simulink library "inp"  (Simulink 8.2)
settings: Solver=ode45  SolverMode=SingleTasking  StartTime=0.0  StopTime=10.0  RelTol=1e-3
undefined symbols (not defined in the file or in its folder): C_TICK_TIME_S, ENUM_FAULTSTATE_FAIL, ENUM_FAULTSTATE_INDETERMINATE, ENUM_FAULTSTATE_PASS, ENUM_KEYSWITCH_ON, FALSE, KINP_ABS1CANCommuOk_flg_ovrdflg, KINP_ABS1CANCommuOk_flg_ovrdval, KINP_ABS1CANPeriodTime_s, KINP_ABS1CANRolCntOk_flg_ovrdflg, KINP_ABS1CANRolCntOk_flg_ovrdval, KINP_ABS2CANChkSumOk_flg_ovrdflg, KINP_ABS2CANChkSumOk_flg_ovrdval, KINP_ABS2CANCommuOk_flg_ovrdflg, KINP_ABS2CANCommuOk_flg_ovrdval, KINP_ABS2CANPeriodTime_s, KINP_ABS2CANRolCntOk_flg_ovrdflg, KINP_ABS2CANRolCntOk_flg_ovrdval, KINP_ABS3CANCommuOk_flg_ovrdflg, KINP_ABS3CANCommuOk_flg_ovrdval, KINP_ABS4CANCommuOk_flg_ovrdflg, KINP_ABS4CANCommuOk_flg_ovrdval, KINP_ABSActive_flg_defval, KINP_ABSActive_flg_invalid, KINP_ABSActive_flg_ovrdflg, KINP_ABSActive_flg_ovrdval, KINP_ABSActive_flg_setvalid, KINP_ABSActive_flg_srvcflg, KINP_ABSActive_flg_srvcval, KINP_ABSCANAlive_flg_ovrdflg, KINP_ABSCANAlive_flg_ovrdval, KINP_ABSCANCommuOk_flg_ovrdflg, KINP_ABSCANCommuOk_flg_ovrdval, KINP_ABSCANValidOk_flg_ovrdflg, KINP_ABSCANValidOk_flg_ovrdval, KINP_ABSFault_flg_defval, KINP_ABSFault_flg_invalid, KINP_ABSFault_flg_ovrdflg, KINP_ABSFault_flg_ovrdval, KINP_ABSFault_flg_setvalid, KINP_ABSFault_flg_srvcflg, KINP_ABSFault_flg_srvcval, KINP_AC1CANCommuOk_flg_ovrdflg, KINP_AC1CANCommuOk_flg_ovrdval, KINP_ACCANAlive_flg_ovrdflg, KINP_ACCANAlive_flg_ovrdval, KINP_ACCANCommuOk_flg_ovrdflg, KINP_ACCANCommuOk_flg_ovrdval, KINP_ACCANValidOk_flg_ovrdflg, KINP_ACCANValidOk_flg_ovrdval, KINP_ACCCancelH_flg_defval, KINP_ACCCancelH_flg_invalid, KINP_ACCCancelH_flg_ovrdflg, KINP_ACCCancelH_flg_ovrdval, KINP_ACCCancelH_flg_setvalid, KINP_ACCCancelH_flg_srvcflg, KINP_ACCCancelH_flg_srvcval, KINP_AccelPedalPosn_pct_defval, KINP_AccelPedalPosn_pct_gain, KINP_AccelPedalPosn_pct_invalid ... (+1866)
libraries: inp x192, hcu_lib x34, simulink x33
(part 21 of 21)

== system inp/INP/InputSignals/SignalProcess/Proc_HMIButtons ==
  VINP_LFCPilotOnH_flg_InHAL : Inport
  VINP_LFCPilotCancelH_flg_InHAL : Inport  Port=2
  VINP_ACCOnH_flg_InHAL : Inport  Port=3
  VINP_ACCCancelH_flg_InHAL : Inport  Port=4
  VINP_ACCResSpdIncH_flg_InHAL : Inport  Port=5
  VINP_ACCSetSpdDecH_flg_InHAL : Inport  Port=6
  VINP_ACCSpdIncH_flg_InHAL : Inport  Port=7
  VINP_ACCSpdDecH_flg_InHAL : Inport  Port=8
  VINP_ACCTimeGapIncH_flg_InHAL : Inport  Port=9
  VINP_ACCTimeGapDecH_flg_InHAL : Inport  Port=10
  VINP_IGSAOnH_flg_InHAL : Inport  Port=11
  VINP_IGSAResSpdIncH_flg_InHAL : Inport  Port=12
  VINP_IGSASetSpdDecH_flg_InHAL : Inport  Port=13
  VINP_IGSASwitchModeH_flg_InHAL : Inport  Port=14
  Detect Change{,1..13} (14 alike) : simulink/Logic and Bit Operations/Detect Change  vinit=0  InputProcessing=Inherited  OutDataTypeStr=uint8
  VINP_LFCPilotOnH_flg_InProc : Outport
  VINP_LFCPilotCancelH_flg_InProc : Outport  Port=2
  VINP_ACCOnH_flg_InProc : Outport  Port=3
  VINP_ACCCancelH_flg_InProc : Outport  Port=4
  VINP_ACCResSpdIncH_flg_InProc : Outport  Port=5
  VINP_ACCSetSpdDecH_flg_InProc : Outport  Port=6
  VINP_ACCSpdIncH_flg_InProc : Outport  Port=7
  VINP_ACCSpdDecH_flg_InProc : Outport  Port=8
  VINP_ACCTimeGapIncH_flg_InProc : Outport  Port=9
  VINP_ACCTimeGapDecH_flg_InProc : Outport  Port=10
  VINP_IGSAOnH_flg_InProc : Outport  Port=11
  VINP_IGSAResSpdIncH_flg_InProc : Outport  Port=12
  VINP_IGSASetSpdDecH_flg_InProc : Outport  Port=13
  VINP_IGSASwitchModeH_flg_InProc : Outport  Port=14
  wires:
  VINP_LFCPilotOnH_flg_InHAL/1 -> Detect Change/1
  Detect Change/1 -> VINP_LFCPilotOnH_flg_InProc/1  "VINP_LFCPilotOnH_flg_InProc"
  VINP_LFCPilotCancelH_flg_InHAL/1 -> Detect Change1/1
  Detect Change1/1 -> VINP_LFCPilotCancelH_flg_InProc/1  "VINP_LFCPilotCancelH_flg_InProc"
  VINP_ACCOnH_flg_InHAL/1 -> Detect Change2/1
  Detect Change2/1 -> VINP_ACCOnH_flg_InProc/1  "VINP_ACCOnH_flg_InProc"
  VINP_ACCCancelH_flg_InHAL/1 -> Detect Change3/1
  Detect Change3/1 -> VINP_ACCCancelH_flg_InProc/1  "VINP_ACCCancelH_flg_InProc"
  VINP_ACCResSpdIncH_flg_InHAL/1 -> Detect Change4/1
  Detect Change4/1 -> VINP_ACCResSpdIncH_flg_InProc/1  "VINP_ACCResSpdIncH_flg_InProc"
  VINP_ACCSetSpdDecH_flg_InHAL/1 -> Detect Change5/1
  Detect Change5/1 -> VINP_ACCSetSpdDecH_flg_InProc/1  "VINP_ACCSetSpdDecH_flg_InProc"
  VINP_ACCSpdIncH_flg_InHAL/1 -> Detect Change6/1
  Detect Change6/1 -> VINP_ACCSpdIncH_flg_InProc/1  "VINP_ACCSpdIncH_flg_InProc"
  VINP_ACCSpdDecH_flg_InHAL/1 -> Detect Change7/1
  Detect Change7/1 -> VINP_ACCSpdDecH_flg_InProc/1  "VINP_ACCSpdDecH_flg_InProc"
  VINP_ACCTimeGapIncH_flg_InHAL/1 -> Detect Change8/1
  Detect Change8/1 -> VINP_ACCTimeGapIncH_flg_InProc/1  "VINP_ACCTimeGapIncH_flg_InProc"
  VINP_ACCTimeGapDecH_flg_InHAL/1 -> Detect Change9/1
  Detect Change9/1 -> VINP_ACCTimeGapDecH_flg_InProc/1  "VINP_ACCTimeGapDecH_flg_InProc"
  VINP_IGSAOnH_flg_InHAL/1 -> Detect Change10/1
  Detect Change10/1 -> VINP_IGSAOnH_flg_InProc/1  "VINP_IGSAOnH_flg_InProc"
  VINP_IGSAResSpdIncH_flg_InHAL/1 -> Detect Change11/1
  Detect Change11/1 -> VINP_IGSAResSpdIncH_flg_InProc/1  "VINP_IGSAResSpdIncH_flg_InProc"
  VINP_IGSASetSpdDecH_flg_InHAL/1 -> Detect Change12/1
  Detect Change12/1 -> VINP_IGSASetSpdDecH_flg_InProc/1  "VINP_IGSASetSpdDecH_flg_InProc"
  VINP_IGSASwitchModeH_flg_InHAL/1 -> Detect Change13/1
  Detect Change13/1 -> VINP_IGSASwitchModeH_flg_InProc/1  "VINP_IGSASwitchModeH_flg_InProc"

== system inp/INP/InputSignals/SignalProcess/Proc_IgnitionActive ==
  VINP_IgnitionVolt_V_InHAL : Inport
  VINP_KeySwitchStatus_enum_InHAL : Inport  Port=2
  Constant : Constant  Value=ENUM_KEYSWITCH_ON
  Constant1 : Constant  Value=KINP_IgnitionActiveVolt_V
  Logical Operator : Logic  Operator=OR  AllPortsSameDT=off  OutDataTypeStr=boolean
  Relational Operator : RelationalOperator  Operator===  OutDataTypeStr=boolean
  Relational Operator1 : RelationalOperator  Operator=>  OutDataTypeStr=boolean
  VINP_IgnitionActive_flg_InProc : Outport
  wires:
  Constant1/1 -> Relational Operator1/2
  Relational Operator1/1 -> Logical Operator/1
  VINP_IgnitionVolt_V_InHAL/1 -> Relational Operator1/1
  Constant/1 -> Relational Operator/2
  Relational Operator/1 -> Logical Operator/2
  VINP_KeySwitchStatus_enum_InHAL/1 -> Relational Operator/1
  Logical Operator/1 -> VINP_IgnitionActive_flg_InProc/1  "VINP_IgnitionActive_flg_InProc"

== system inp/INP/InputSignals/SignalProcess/Proc_LaneEstimator ==
  VINP_AppTime_ms_InProc : Inport
  Task_Time_s : Inport  Port=2
  VINP_YawRate_radps_InHAL : Inport  Port=3
  VINP_VehSpd_mps_InHAL : Inport  Port=4
  VINP_LaneC0L_m_InHAL : Inport  Port=5
  VINP_LaneC1L_rad_InHAL : Inport  Port=6
  VINP_LaneC2L_rat_InHAL : Inport  Port=7
  VINP_LaneC3L_rat_InHAL : Inport  Port=8
  VINP_LaneC0R_m_InHAL : Inport  Port=9
  VINP_LaneC1R_rad_InHAL : Inport  Port=10
  VINP_LaneC2R_rat_InHAL : Inport  Port=11
  VINP_LaneC3R_rat_InHAL : Inport  Port=12
  VINP_LaneQualityL_enum_InHAL : Inport  Port=13
  VINP_LaneQualityR_enum_InHAL : Inport  Port=14
  Ground{,1..7} (8 alike) : Ground
  Terminator{,1..13} (14 alike) : Terminator
  VINP_LaneC0LKalF_m_InProc : Outport
  VINP_LaneC1LKalF_rad_InProc : Outport  Port=2
  VINP_LaneC2LKalF_rat_InProc : Outport  Port=3
  VINP_LaneC3LKalF_rat_InProc : Outport  Port=4
  VINP_LaneC0RKalF_m_InProc : Outport  Port=5
  VINP_LaneC1RKalF_rad_InProc : Outport  Port=6
  VINP_LaneC2RKalF_rat_InProc : Outport  Port=7
  VINP_LaneC3RKalF_rat_InProc : Outport  Port=8
  wires:
  Ground6/1 -> VINP_LaneC3LKalF_rat_InProc/1  "VINP_LaneC3LKalF_rat_InProc"
  Ground7/1 -> VINP_LaneC3RKalF_rat_InProc/1  "VINP_LaneC3RKalF_rat_InProc"
  Ground/1 -> VINP_LaneC0LKalF_m_InProc/1  "VINP_LaneC0LKalF_m_InProc"
  Ground1/1 -> VINP_LaneC1LKalF_rad_InProc/1  "VINP_LaneC1LKalF_rad_InProc"
  Ground2/1 -> VINP_LaneC2LKalF_rat_InProc/1  "VINP_LaneC2LKalF_rat_InProc"
  Ground3/1 -> VINP_LaneC0RKalF_m_InProc/1  "VINP_LaneC0RKalF_m_InProc"
  Ground4/1 -> VINP_LaneC1RKalF_rad_InProc/1  "VINP_LaneC1RKalF_rad_InProc"
  Ground5/1 -> VINP_LaneC2RKalF_rat_InProc/1  "VINP_LaneC2RKalF_rat_InProc"
  VINP_AppTime_ms_InProc/1 -> Terminator/1
  Task_Time_s/1 -> Terminator1/1
  VINP_YawRate_radps_InHAL/1 -> Terminator2/1
  VINP_VehSpd_mps_InHAL/1 -> Terminator3/1
  VINP_LaneQualityL_enum_InHAL/1 -> Terminator4/1
  VINP_LaneQualityR_enum_InHAL/1 -> Terminator5/1
  VINP_LaneC0L_m_InHAL/1 -> Terminator6/1
  VINP_LaneC1L_rad_InHAL/1 -> Terminator7/1
  VINP_LaneC2L_rat_InHAL/1 -> Terminator8/1
  VINP_LaneC3L_rat_InHAL/1 -> Terminator9/1
  VINP_LaneC0R_m_InHAL/1 -> Terminator10/1
  VINP_LaneC1R_rad_InHAL/1 -> Terminator11/1
  VINP_LaneC2R_rat_InHAL/1 -> Terminator12/1
  VINP_LaneC3R_rat_InHAL/1 -> Terminator13/1

== system inp/INP/InputSignals/SignalProcess/Proc_OtherHMISwitches ==
  VINP_RearFogSwOn_flg_InHAL : Inport
  VINP_RearFogSwOnValid_flg_InHAL : Inport  Port=2
  Data Type Conversion : DataTypeConversion  OutDataTypeStr=boolean
  RearFogOnSwitchLatch : Stateflow chart  TreatAsAtomicUnit=on
  VINP_RearFogSwOn_flg_InProc : Outport
  VINP_RearFogSwOnValid_flg_InProc : Outport  Port=2
  wires:
  VINP_RearFogSwOn_flg_InHAL/1 -> RearFogOnSwitchLatch/1(VINP_RearFogSwOn_flg_InHAL)
  RearFogOnSwitchLatch/1(VINP_RearFogSwOn_flg_InProc) -> VINP_RearFogSwOn_flg_InProc/1
  Data Type Conversion/1 -> VINP_RearFogSwOnValid_flg_InProc/1  "VINP_RearFogSwOnValid_flg_InProc"
  VINP_RearFogSwOnValid_flg_InHAL/1 -> Data Type Conversion/1

== system inp/INP/InputSignals/SignalProcess/Proc_SteerAngleUnit ==
  VINP_SteerAngle_deg_InHAL : Inport
  deg2rad : hcu_lib/deg2rad
  VINP_SteerAngle_rad_InProc : Outport
  wires:
  VINP_SteerAngle_deg_InHAL/1 -> deg2rad/1
  deg2rad/1 -> VINP_SteerAngle_rad_InProc/1  "VINP_SteerAngle_rad_InProc"

== system inp/INP/InputSignals/SignalProcess/Proc_SteerSpeedCal ==
  VINP_SteerAngle_deg_InHAL : Inport
  VINP_SteerAngleValid_flg_InHAL : Inport  Port=2
  CAN_Update_flag : Stateflow chart  TreatAsAtomicUnit=on
  Data Type Conversion : DataTypeConversion
  Detect Change : simulink/Logic and Bit Operations/Detect Change  vinit=0  InputProcessing=Inherited  OutDataTypeStr=boolean
  SteerSpeedCalculation : Subsystem
  SteerSpeedCalculation1 : Subsystem
  StrRate_SW : Constant  Value=0  OutDataTypeStr=boolean
  StrRate_calculation : Stateflow chart  TreatAsAtomicUnit=on
  Switch : Switch
  Trigger signal generator : Subsystem  TreatAsAtomicUnit=on
  Unit Delay1 : UnitDelay  SampleTime=-1
  VINP_SteerSpeed_degps_InProc : Outport
  VINP_SteerSpeedValid_flg_InProc : Outport  Port=2
  wires:
  Trigger signal generator/1(Trigger_signal) -> SteerSpeedCalculation1/Trigger, SteerSpeedCalculation/Trigger
  Data Type Conversion/1 -> VINP_SteerSpeedValid_flg_InProc/1  "VINP_SteerSpeedValid_flg_InProc"
  VINP_SteerAngle_deg_InHAL/1 -> SteerSpeedCalculation1/1(VSTR_StrAngReq_deg), SteerSpeedCalculation/1(In1)
  VINP_SteerAngleValid_flg_InHAL/1 -> Data Type Conversion/1
  StrRate_calculation/1(StrRate) -> Switch/3, Unit Delay1/1
  CAN_Update_flag/1(CAN_flag) -> StrRate_calculation/1(CAN_flag)  "CAN_flag"
  Detect Change/1 -> CAN_Update_flag/1(AngleChg)
  SteerSpeedCalculation/1(StrAng) -> Detect Change/1, StrRate_calculation/2(StrAng)  "StrAng"
  SteerSpeedCalculation/2(StrAng1) -> StrRate_calculation/3(StrAng1)  "StrAng1"
  SteerSpeedCalculation/3(StrAng2) -> StrRate_calculation/4(StrAng2)  "StrAng2"
  SteerSpeedCalculation/4(StrAng3) -> StrRate_calculation/5(StrAng3)  "StrAng3"
  SteerSpeedCalculation/5(StrAng4) -> StrRate_calculation/6(StrAng4)  "StrAng4"
  Unit Delay1/1 -> StrRate_calculation/7(StrRateLast)  "StrRateLast"
  Switch/1 -> VINP_SteerSpeed_degps_InProc/1  "VINP_SteerSpeed_degps_InProc"
  SteerSpeedCalculation1/1(VSTR_StrRateReq_degps) -> Switch/1
  StrRate_SW/1 -> Switch/2

== system inp/INP/InputSignals/SignalProcess/Proc_SteeringWheelButtons ==
  VINP_ACCOnVCU_flg_InHAL : Inport
  VINP_ACCOnVCUValid_flg_InHAL : Inport  Port=2
  VINP_ACCTimeGapDecVCU_flg_InHAL : Inport  Port=3
  VINP_ACCTimeGapDecVCUValid_flg_InHAL : Inport  Port=4
  VINP_LFCOnVCU_flg_InHAL : Inport  Port=5
  VINP_LFCOnVCUValid_flg_InHAL : Inport  Port=6
  Data Type Conversion{,1..3} (4 alike) : DataTypeConversion  OutDataTypeStr=boolean
  Detect Increase : simulink/Logic and Bit Operations/Detect Increase  vinit=0.0  InputProcessing=Inherited  OutDataTypeStr=boolean
  Detect Increase1 : simulink/Logic and Bit Operations/Detect Increase  vinit=0.0  InputProcessing=Inherited  OutDataTypeStr=boolean
  VINP_ACCOnVCU_flg_InProc : Outport
  VINP_ACCOnVCUValid_flg_InProc : Outport  Port=2
  VINP_ACCDistVCU_flg_InProc : Outport  Port=3
  VINP_ACCDistVCUValid_flg_InProc : Outport  Port=4
  VINP_LFCOnVCU_flg_InProc : Outport  Port=5
  VINP_LFCOnVCUValid_flg_InProc : Outport  Port=6
  wires:
  Data Type Conversion/1 -> VINP_ACCOnVCUValid_flg_InProc/1  "VINP_ACCOnVCUValid_flg_InProc"
  VINP_ACCOnVCUValid_flg_InHAL/1 -> Data Type Conversion/1
  Detect Increase1/1 -> VINP_ACCOnVCU_flg_InProc/1  "VINP_ACCOnVCU_flg_InProc"
  VINP_ACCOnVCU_flg_InHAL/1 -> Detect Increase1/1
  VINP_ACCTimeGapDecVCU_flg_InHAL/1 -> Data Type Conversion1/1
  VINP_ACCTimeGapDecVCUValid_flg_InHAL/1 -> Data Type Conversion2/1
  Data Type Conversion1/1 -> VINP_ACCDistVCU_flg_InProc/1  "VINP_ACCDistVCU_flg_InProc"
  Data Type Conversion2/1 -> VINP_ACCDistVCUValid_flg_InProc/1  "VINP_ACCDistVCUValid_flg_InProc"
  Data Type Conversion3/1 -> VINP_LFCOnVCUValid_flg_InProc/1  "VINP_LFCOnVCUValid_flg_InProc"
  VINP_LFCOnVCUValid_flg_InHAL/1 -> Data Type Conversion3/1
  Detect Increase/1 -> VINP_LFCOnVCU_flg_InProc/1  "VINP_LFCOnVCU_flg_InProc"
  VINP_LFCOnVCU_flg_InHAL/1 -> Detect Increase/1

== system inp/INP/InputSignals/SignalProcess/Proc_VehicleSpeedUnit ==
  VINP_VehSpd_kph_InHAL : Inport
  kph2mps : hcu_lib/kph2mps
  VINP_VehSpd_mps_InProc : Outport
  wires:
  kph2mps/1 -> VINP_VehSpd_mps_InProc/1  "VINP_VehSpd_mps_InProc"
  VINP_VehSpd_kph_InHAL/1 -> kph2mps/1

== system inp/INP/InputSignals/SignalProcess/Proc_YawRateUnit ==
  VINP_YawRate_degps_InHAL : Inport
  deg2rad : hcu_lib/deg2rad
  VINP_YawRate_radps_InProc : Outport
  wires:
  deg2rad/1 -> VINP_YawRate_radps_InProc/1  "VINP_YawRate_radps_InProc"
  VINP_YawRate_degps_InHAL/1 -> deg2rad/1

== system inp/INP/METER_signals/MeterVehSpd/conv_gain_offset ==
  in : Inport
  Product : Product  RndMeth=Zero
  Sum : Sum
  gain : Constant  Value=KINP_MeterVehSpd_kph_gain
  offset : Constant  Value=KINP_MeterVehSpd_kph_offset
  out : Outport
  wires:
  offset/1 -> Sum/2
  Product/1 -> Sum/1
  gain/1 -> Product/2
  in/1 -> Product/1
  Sum/1 -> out/1

== system inp/INP/METER_signals/MeterVehSpd/min_max_limit ==
  in : Inport
  MinMax10 : MinMax  Function=max  Inputs=2
  MinMax5 : MinMax  Inputs=2
  maxval : Constant  Value=KINP_MeterVehSpd_kph_maxval
  minval : Constant  Value=KINP_MeterVehSpd_kph_minval
  out : Outport
  wires:
  minval/1 -> MinMax10/2
  maxval/1 -> MinMax5/2
  MinMax10/1 -> out/1
  in/1 -> MinMax5/1
  MinMax5/1 -> MinMax10/1

== system inp/INP/METER_signals/OdoMeter/conv_gain_offset ==
  in : Inport
  Product : Product  RndMeth=Zero
  Sum : Sum
  gain : Constant  Value=KINP_OdoMeter_km_gain
  offset : Constant  Value=KINP_OdoMeter_km_offset
  out : Outport
  wires:
  Sum/1 -> out/1
  in/1 -> Product/1
  gain/1 -> Product/2
  Product/1 -> Sum/1
  offset/1 -> Sum/2

== system inp/INP/METER_signals/OdoMeter/min_max_limit ==
  in : Inport
  MinMax10 : MinMax  Function=max  Inputs=2
  MinMax5 : MinMax  Inputs=2
  maxval : Constant  Value=KINP_OdoMeter_km_maxval
  minval : Constant  Value=KINP_OdoMeter_km_minval
  out : Outport
  wires:
  MinMax5/1 -> MinMax10/1
  in/1 -> MinMax5/1
  MinMax10/1 -> out/1
  maxval/1 -> MinMax5/2
  minval/1 -> MinMax10/2

== system inp/INP/TCM_signals/TMTurbineSpd/conv_gain_offset ==
  in : Inport
  Product : Product  RndMeth=Zero
  Sum : Sum
  gain : Constant  Value=KINP_TMTurbineSpd_rpm_gain
  offset : Constant  Value=KINP_TMTurbineSpd_rpm_offset
  out : Outport
  wires:
  Sum/1 -> out/1
  in/1 -> Product/1
  gain/1 -> Product/2
  Product/1 -> Sum/1
  offset/1 -> Sum/2

== system inp/INP/TCM_signals/TMTurbineSpd/min_max_limit ==
  in : Inport
  MinMax10 : MinMax  Function=max  Inputs=2
  MinMax5 : MinMax  Inputs=2
  maxval : Constant  Value=KINP_TMTurbineSpd_rpm_maxval
  minval : Constant  Value=KINP_TMTurbineSpd_rpm_minval
  out : Outport
  wires:
  MinMax5/1 -> MinMax10/1
  in/1 -> MinMax5/1
  MinMax10/1 -> out/1
  maxval/1 -> MinMax5/2
  minval/1 -> MinMax10/2

== system inp/INP/VCU_signals/AckCurBrakDecReq/conv_gain_offset ==
  in : Inport
  Product : Product  RndMeth=Zero
  Sum : Sum
  gain : Constant  Value=KINP_AckCurBrakDecReq_mps2_gain
  offset : Constant  Value=KINP_AckCurBrakDecReq_mps2_offset
  out : Outport
  wires:
  Sum/1 -> out/1
  in/1 -> Product/1
  gain/1 -> Product/2
  Product/1 -> Sum/1
  offset/1 -> Sum/2

== system inp/INP/VCU_signals/AckCurBrakDecReq/min_max_limit ==
  in : Inport
  MinMax10 : MinMax  Function=max  Inputs=2
  MinMax5 : MinMax  Inputs=2
  maxval : Constant  Value=KINP_AckCurBrakDecReq_mps2_maxval
  minval : Constant  Value=KINP_AckCurBrakDecReq_mps2_minval
  out : Outport
  wires:
  MinMax5/1 -> MinMax10/1
  in/1 -> MinMax5/1
  MinMax10/1 -> out/1
  maxval/1 -> MinMax5/2
  minval/1 -> MinMax10/2

== system inp/INP/VCU_signals/AckCurPTTqReq/conv_gain_offset ==
  in : Inport
  Product : Product  RndMeth=Zero
  Sum : Sum
  gain : Constant  Value=KINP_AckCurPTTqReq_Nm_gain
  offset : Constant  Value=KINP_AckCurPTTqReq_Nm_offset
  out : Outport
  wires:
  offset/1 -> Sum/2
  Product/1 -> Sum/1
  gain/1 -> Product/2
  in/1 -> Product/1
  Sum/1 -> out/1

== system inp/INP/VCU_signals/AckCurPTTqReq/min_max_limit ==
  in : Inport
  MinMax10 : MinMax  Function=max  Inputs=2
  MinMax5 : MinMax  Inputs=2
  maxval : Constant  Value=KINP_AckCurPTTqReq_Nm_maxval
  minval : Constant  Value=KINP_AckCurPTTqReq_Nm_minval
  out : Outport
  wires:
  minval/1 -> MinMax10/2
  maxval/1 -> MinMax5/2
  MinMax10/1 -> out/1
  in/1 -> MinMax5/1
  MinMax5/1 -> MinMax10/1

== system inp/INP/VCU_signals/AckCurVehDec/conv_gain_offset ==
  in : Inport
  Product : Product  RndMeth=Zero
  Sum : Sum
  gain : Constant  Value=KINP_AckCurVehDec_mps2_gain
  offset : Constant  Value=KINP_AckCurVehDec_mps2_offset
  out : Outport
  wires:
  offset/1 -> Sum/2
  Product/1 -> Sum/1
  gain/1 -> Product/2
  in/1 -> Product/1
  Sum/1 -> out/1

== system inp/INP/VCU_signals/AckCurVehDec/min_max_limit ==
  in : Inport
  MinMax10 : MinMax  Function=max  Inputs=2
  MinMax5 : MinMax  Inputs=2
  maxval : Constant  Value=KINP_AckCurVehDec_mps2_maxval
  minval : Constant  Value=KINP_AckCurVehDec_mps2_minval
  out : Outport
  wires:
  minval/1 -> MinMax10/2
  maxval/1 -> MinMax5/2
  MinMax10/1 -> out/1
  in/1 -> MinMax5/1
  MinMax5/1 -> MinMax10/1

== system inp/INP/VCU_signals/CurDriverTqReq/conv_gain_offset ==
  in : Inport
  Product : Product  RndMeth=Zero
  Sum : Sum
  gain : Constant  Value=KINP_CurDriverTqReq_Nm_gain
  offset : Constant  Value=KINP_CurDriverTqReq_Nm_offset
  out : Outport
  wires:
  Sum/1 -> out/1
  in/1 -> Product/1
  gain/1 -> Product/2
  Product/1 -> Sum/1
  offset/1 -> Sum/2

== system inp/INP/VCU_signals/CurDriverTqReq/min_max_limit ==
  in : Inport
  MinMax10 : MinMax  Function=max  Inputs=2
  MinMax5 : MinMax  Inputs=2
  maxval : Constant  Value=KINP_CurDriverTqReq_Nm_maxval
  minval : Constant  Value=KINP_CurDriverTqReq_Nm_minval
  out : Outport
  wires:
  MinMax5/1 -> MinMax10/1
  in/1 -> MinMax5/1
  MinMax10/1 -> out/1
  maxval/1 -> MinMax5/2
  minval/1 -> MinMax10/2

== system inp/INP/VCU_signals/ESCBattVolt/conv_gain_offset ==
  in : Inport
  Product : Product  RndMeth=Zero
  Sum : Sum
  gain : Constant  Value=KINP_ESCBattVolt_V_gain
  offset : Constant  Value=KINP_ESCBattVolt_V_offset
  out : Outport
  wires:
  Sum/1 -> out/1
  in/1 -> Product/1
  gain/1 -> Product/2
  Product/1 -> Sum/1
  offset/1 -> Sum/2

== system inp/INP/VCU_signals/ESCBattVolt/min_max_limit ==
  in : Inport
  MinMax10 : MinMax  Function=max  Inputs=2
  MinMax5 : MinMax  Inputs=2
  maxval : Constant  Value=KINP_ESCBattVolt_V_maxval
  minval : Constant  Value=KINP_ESCBattVolt_V_minval
  out : Outport
  wires:
  MinMax5/1 -> MinMax10/1
  in/1 -> MinMax5/1
  MinMax10/1 -> out/1
  maxval/1 -> MinMax5/2
  minval/1 -> MinMax10/2

== system inp/INP/VCU_signals/HWAccelPedalPosn/conv_gain_offset ==
  in : Inport
  Product : Product  RndMeth=Zero
  Sum : Sum
  gain : Constant  Value=KINP_HWAccelPedalPosn_pct_gain
  offset : Constant  Value=KINP_HWAccelPedalPosn_pct_offset
  out : Outport
  wires:
  offset/1 -> Sum/2
  Product/1 -> Sum/1
  gain/1 -> Product/2
  in/1 -> Product/1
  Sum/1 -> out/1

== system inp/INP/VCU_signals/HWAccelPedalPosn/min_max_limit ==
  in : Inport
  MinMax10 : MinMax  Function=max  Inputs=2
  MinMax5 : MinMax  Inputs=2
  maxval : Constant  Value=KINP_HWAccelPedalPosn_pct_maxval
  minval : Constant  Value=KINP_HWAccelPedalPosn_pct_minval
  out : Outport
  wires:
  minval/1 -> MinMax10/2
  maxval/1 -> MinMax5/2
  MinMax10/1 -> out/1
  in/1 -> MinMax5/1
  MinMax5/1 -> MinMax10/1

== system inp/INP/HWIO_signals/BatteryVolt/ad_signal_process/sig_valid_check ==
  input_signal_filt : Inport
  adc_fault : Inport  Port=2
  vref_fault : Inport  Port=3
  range_high_ifault_flg : Inport  Port=4
  range_low_ifault_flg : Inport  Port=5
  def_value : Inport  Port=6
  Active_fault_flg : Inport  Port=7
  Logical Operator1 : Logic  Operator=OR  Inputs=4  AllPortsSameDT=off  OutDataTypeStr=boolean
  Logical Operator3 : Logic  Operator=OR  AllPortsSameDT=off  OutDataTypeStr=boolean
  Relational Operator{,1..3} (4 alike) : RelationalOperator  Operator===  OutDataTypeStr=boolean
  Switch{,1..5} (6 alike) : Switch  Criteria=u2 ~= 0
  Unit Delay : UnitDelay  SampleTime=-1
  zero{,1,4,8} (4 alike) : Constant  Tag=struct('comment',struct('text','','placement',1),'output',struct('width',1,'name','$S_$B','unit','','type','Int16','offset',0,'max',NaN,'min',NaN,'freeze',1,'lsb',1,'arb',0,'class','default','address','','description','','value','0','tolerance',1,'show',0),'date',731455.58675544)  Value=ENUM_FAULTSTATE_FAIL
  zero{2..3,6..7} (4 alike) : Constant  Tag=struct('comment',struct('text','','placement',1),'output',struct('width',1,'name','$S_$B','unit','','type','Int16','offset',0,'max',NaN,'min',NaN,'freeze',1,'lsb',1,'arb',0,'class','default','address','','description','','value','0','tolerance',1,'show',0),'date',731455.58675544)  Value=ENUM_FAULTSTATE_INDETERMINATE
  zero5 : Constant  Tag=struct('comment',struct('text','','placement',1),'output',struct('width',1,'name','$S_$B','unit','','type','Int16','offset',0,'max',NaN,'min',NaN,'freeze',1,'lsb',1,'arb',0,'class','default','address','','description','','value','0','tolerance',1,'show',0),'date',731455.58675544)  Value=ENUM_FAULTSTATE_PASS
  zero9 : Constant  Tag=struct('comment',struct('text','','placement',1),'output',struct('width',1,'name','$S_$B','unit','','type','Int16','offset',0,'max',NaN,'min',NaN,'freeze',1,'lsb',1,'arb',0,'class','default','address','','description','','value','0','tolerance',1,'show',0),'date',731455.58675544)  Value=ENUM_FAULTSTATE_PASS
  range_high_flt : Outport
  range_low_flt : Outport  Port=2
  fault_flg : Outport  Port=3
  valid_signal : Outport  Port=4
  wires:
  Unit Delay/1 -> Switch1/1
  [fault_flg] -> Switch/2
  Switch/1 -> Unit Delay/1, valid_signal/1
  Switch1/1 -> Switch/3
  def_value/1 -> Switch/1
  range_high_ifault_flg/1 -> [inst_high_fault]
  input_signal_filt/1 -> Switch1/3
  Logical Operator1/1 -> Switch1/2  "ifault_flg"
  range_low_ifault_flg/1 -> [inst_low_fault]
  adc_fault/1 -> Relational Operator/1, [adc_fault]
  zero/1 -> Relational Operator/2
  zero1/1 -> Relational Operator1/2
  vref_fault/1 -> Relational Operator1/1, [vref_fault]
  Relational Operator/1 -> [inst_adc_fault]
  Relational Operator1/1 -> [inst_vref_fault]
  Active_fault_flg/1 -> fault_flg/1, [fault_flg]  "Active_fault_flg"
  [inst_adc_fault] -> Logical Operator1/1
  [inst_vref_fault] -> Logical Operator1/2
  [inst_high_fault] -> Logical Operator1/3
  [inst_low_fault] -> Logical Operator1/4
  zero2/1 -> Relational Operator2/2
  [inst_high_fault] -> Switch3/2
  Switch3/1 -> Switch2/3
  zero3/1 -> Switch2/1
  [adc_fault] -> Relational Operator2/1
  zero4/1 -> Switch3/1
  zero5/1 -> Switch3/3
  Switch2/1 -> range_high_flt/1
  zero6/1 -> Relational Operator3/2
  [vref_fault] -> Relational Operator3/1
  Relational Operator2/1 -> Logical Operator3/1
  Relational Operator3/1 -> Logical Operator3/2
  [indeterminate] -> Switch2/2
  Logical Operator3/1 -> [indeterminate]
  [inst_low_fault] -> Switch5/2
  Switch5/1 -> Switch4/3
  zero7/1 -> Switch4/1
  zero8/1 -> Switch5/1
  zero9/1 -> Switch5/3
  [indeterminate] -> Switch4/2
  Switch4/1 -> range_low_flt/1

== system inp/INP/HWIO_signals/BatteryVolt/ad_signal_process/signal_filter ==
  raw_input : Inport
  Tc : Inport  Port=2
  Ts : Inport  Port=3
  FirstOrderFilter : hcu_lib/FirstOrderFilter  SystemSampleTime=-1
  IC : UnitDelay  Tag=struct('comment',struct('text','','placement',1),'logdata',struct('maxnumvals',inf,'plotchannels',[],'stepsize',0,'downsampling',1,'loggingmode',3,'channels',1),'output',struct('width',1,'name','$S_$B','unit','','type','Int16','offset',0,'max',NaN,'min',NaN,'freeze',1,'lsb',1,'arb',0,'class','default','address','','description','','autoscalingmode',1,'lowerheadroom',1,'upperheadroom',1,'headroomunit','bit','checkmin',0,'checkmax',0,'show',0,'initial','2'),'state',0,'dt','-1','date',731455.586925799)  SampleTime=-1
  Switch1 : Switch  Tag=struct('comment',struct('text','','placement',1),'logdata',struct('maxnumvals',inf,'plotchannels',[],'stepsize',0,'downsampling',1,'loggingmode',3,'channels',1),'output',struct('width',1,'name','$S_$B','unit','','type','Int32Bin15','offset',0,'max',65535,'min',-65536,'freeze',1,'lsb',2^-15,'arb',0,'class','default','address','','description','','autoscalingmode',1,'lowerheadroom',1,'upperheadroom',1,'headroomunit','bit','checkmin',0,'checkmax',0,'show',0),'threshold',struct('width',1,'name','$S_$B_threshold','unit','','type','Int16','offset',0,'max',NaN,'min',NaN,'freeze',1,'lsb',1,'arb',0,'class','default','address','','description','','value','1','scalar',0),'date',731455.58657044)  Threshold=0.5  InputSameDT=on
  zero : Constant  Tag=struct('comment',struct('text','','placement',1),'output',struct('width',1,'name','$S_$B','unit','','type','Int16','offset',0,'max',NaN,'min',NaN,'freeze',1,'lsb',1,'arb',0,'class','default','address','','description','','value','0','tolerance',1,'show',0),'date',731455.58675544)  Value=TRUE
  zero1 : Constant  Tag=struct('comment',struct('text','','placement',1),'output',struct('width',1,'name','$S_$B','unit','','type','Int16','offset',0,'max',NaN,'min',NaN,'freeze',1,'lsb',1,'arb',0,'class','default','address','','description','','value','0','tolerance',1,'show',0),'date',731455.58675544)  Value=0  OutDataTypeStr=single
  raw_input_filt : Outport
  wires:
  zero1/1 -> Switch1/3
  Switch1/1 -> FirstOrderFilter/2
  FirstOrderFilter/1 -> raw_input_filt/1
  IC/1 -> Switch1/2
  Ts/1 -> FirstOrderFilter/3
  Tc/1 -> Switch1/1
  raw_input/1 -> FirstOrderFilter/1
  zero/1 -> IC/1

== system inp/INP/HWIO_signals/IgnitionVolt/ad_signal_process/sig_valid_check ==
  input_signal_filt : Inport
  adc_fault : Inport  Port=2
  vref_fault : Inport  Port=3
  range_high_ifault_flg : Inport  Port=4
  range_low_ifault_flg : Inport  Port=5
  def_value : Inport  Port=6
  Active_fault_flg : Inport  Port=7
  Logical Operator1 : Logic  Operator=OR  Inputs=4  AllPortsSameDT=off  OutDataTypeStr=boolean
  Logical Operator3 : Logic  Operator=OR  AllPortsSameDT=off  OutDataTypeStr=boolean
  Relational Operator{,1..3} (4 alike) : RelationalOperator  Operator===  OutDataTypeStr=boolean
  Switch{,1..5} (6 alike) : Switch  Criteria=u2 ~= 0
  Unit Delay : UnitDelay  SampleTime=-1
  zero{,1,4,8} (4 alike) : Constant  Tag=struct('comment',struct('text','','placement',1),'output',struct('width',1,'name','$S_$B','unit','','type','Int16','offset',0,'max',NaN,'min',NaN,'freeze',1,'lsb',1,'arb',0,'class','default','address','','description','','value','0','tolerance',1,'show',0),'date',731455.58675544)  Value=ENUM_FAULTSTATE_FAIL
  zero{2..3,6..7} (4 alike) : Constant  Tag=struct('comment',struct('text','','placement',1),'output',struct('width',1,'name','$S_$B','unit','','type','Int16','offset',0,'max',NaN,'min',NaN,'freeze',1,'lsb',1,'arb',0,'class','default','address','','description','','value','0','tolerance',1,'show',0),'date',731455.58675544)  Value=ENUM_FAULTSTATE_INDETERMINATE
  zero5 : Constant  Tag=struct('comment',struct('text','','placement',1),'output',struct('width',1,'name','$S_$B','unit','','type','Int16','offset',0,'max',NaN,'min',NaN,'freeze',1,'lsb',1,'arb',0,'class','default','address','','description','','value','0','tolerance',1,'show',0),'date',731455.58675544)  Value=ENUM_FAULTSTATE_PASS
  zero9 : Constant  Tag=struct('comment',struct('text','','placement',1),'output',struct('width',1,'name','$S_$B','unit','','type','Int16','offset',0,'max',NaN,'min',NaN,'freeze',1,'lsb',1,'arb',0,'class','default','address','','description','','value','0','tolerance',1,'show',0),'date',731455.58675544)  Value=ENUM_FAULTSTATE_PASS
  range_high_flt : Outport
  range_low_flt : Outport  Port=2
  fault_flg : Outport  Port=3
  valid_signal : Outport  Port=4
  wires:
  Switch4/1 -> range_low_flt/1
  [indeterminate] -> Switch4/2
  zero9/1 -> Switch5/3
  zero8/1 -> Switch5/1
  zero7/1 -> Switch4/1
  Switch5/1 -> Switch4/3
  [inst_low_fault] -> Switch5/2
  Logical Operator3/1 -> [indeterminate]
  [indeterminate] -> Switch2/2
  Relational Operator3/1 -> Logical Operator3/2
  Relational Operator2/1 -> Logical Operator3/1
  [vref_fault] -> Relational Operator3/1
  zero6/1 -> Relational Operator3/2
  Switch2/1 -> range_high_flt/1
  zero5/1 -> Switch3/3
  zero4/1 -> Switch3/1
  [adc_fault] -> Relational Operator2/1
  zero3/1 -> Switch2/1
  Switch3/1 -> Switch2/3
  [inst_high_fault] -> Switch3/2
  zero2/1 -> Relational Operator2/2
  [inst_low_fault] -> Logical Operator1/4
  [inst_high_fault] -> Logical Operator1/3
  [inst_vref_fault] -> Logical Operator1/2
  [inst_adc_fault] -> Logical Operator1/1
  Active_fault_flg/1 -> [fault_flg], fault_flg/1  "Active_fault_flg"
  Relational Operator1/1 -> [inst_vref_fault]
  Relational Operator/1 -> [inst_adc_fault]
  vref_fault/1 -> [vref_fault], Relational Operator1/1
  zero1/1 -> Relational Operator1/2
  zero/1 -> Relational Operator/2
  adc_fault/1 -> [adc_fault], Relational Operator/1
  range_low_ifault_flg/1 -> [inst_low_fault]
  Logical Operator1/1 -> Switch1/2  "ifault_flg"
  input_signal_filt/1 -> Switch1/3
  range_high_ifault_flg/1 -> [inst_high_fault]
  def_value/1 -> Switch/1
  Switch1/1 -> Switch/3
  Switch/1 -> valid_signal/1, Unit Delay/1
  [fault_flg] -> Switch/2
  Unit Delay/1 -> Switch1/1

== system inp/INP/HWIO_signals/IgnitionVolt/ad_signal_process/signal_filter ==
  raw_input : Inport
  Tc : Inport  Port=2
  Ts : Inport  Port=3
  FirstOrderFilter : hcu_lib/FirstOrderFilter  SystemSampleTime=-1
  IC : UnitDelay  Tag=struct('comment',struct('text','','placement',1),'logdata',struct('maxnumvals',inf,'plotchannels',[],'stepsize',0,'downsampling',1,'loggingmode',3,'channels',1),'output',struct('width',1,'name','$S_$B','unit','','type','Int16','offset',0,'max',NaN,'min',NaN,'freeze',1,'lsb',1,'arb',0,'class','default','address','','description','','autoscalingmode',1,'lowerheadroom',1,'upperheadroom',1,'headroomunit','bit','checkmin',0,'checkmax',0,'show',0,'initial','2'),'state',0,'dt','-1','date',731455.586925799)  SampleTime=-1
  Switch1 : Switch  Tag=struct('comment',struct('text','','placement',1),'logdata',struct('maxnumvals',inf,'plotchannels',[],'stepsize',0,'downsampling',1,'loggingmode',3,'channels',1),'output',struct('width',1,'name','$S_$B','unit','','type','Int32Bin15','offset',0,'max',65535,'min',-65536,'freeze',1,'lsb',2^-15,'arb',0,'class','default','address','','description','','autoscalingmode',1,'lowerheadroom',1,'upperheadroom',1,'headroomunit','bit','checkmin',0,'checkmax',0,'show',0),'threshold',struct('width',1,'name','$S_$B_threshold','unit','','type','Int16','offset',0,'max',NaN,'min',NaN,'freeze',1,'lsb',1,'arb',0,'class','default','address','','description','','value','1','scalar',0),'date',731455.58657044)  Threshold=0.5  InputSameDT=on
  zero : Constant  Tag=struct('comment',struct('text','','placement',1),'output',struct('width',1,'name','$S_$B','unit','','type','Int16','offset',0,'max',NaN,'min',NaN,'freeze',1,'lsb',1,'arb',0,'class','default','address','','description','','value','0','tolerance',1,'show',0),'date',731455.58675544)  Value=TRUE
  zero1 : Constant  Tag=struct('comment',struct('text','','placement',1),'output',struct('width',1,'name','$S_$B','unit','','type','Int16','offset',0,'max',NaN,'min',NaN,'freeze',1,'lsb',1,'arb',0,'class','default','address','','description','','value','0','tolerance',1,'show',0),'date',731455.58675544)  Value=0  OutDataTypeStr=single
  raw_input_filt : Outport
  wires:
  zero/1 -> IC/1
  raw_input/1 -> FirstOrderFilter/1
  Tc/1 -> Switch1/1
  Ts/1 -> FirstOrderFilter/3
  IC/1 -> Switch1/2
  FirstOrderFilter/1 -> raw_input_filt/1
  Switch1/1 -> FirstOrderFilter/2
  zero1/1 -> Switch1/3

== system inp/INP/InputSignals/SignalProcess/Proc_SteerSpeedCal/SteerSpeedCalculation ==
  note: deg
  In1 : Inport
  Trigger : TriggerPort
  Abs : Abs
  Compare To Zero : simulink/Logic and Bit Operations/Compare To Zero  relop=>=  OutDataTypeStr=boolean
  Constant : Constant  Value=single(1)
  Constant1 : Constant  Value=single(-1)
  Constant2 : Constant  Value=KINP_SteerSpeedCalRate_s
  Constant3 : Constant  Value=KINP_SteerSpeedCalRate_s
  Constant4 : Constant  Value=KINP_SteerSpeedFltConst_s
  Divide : Product  Inputs=*/
  Product : Product
  Sum : Sum  Inputs=+-
  Switch : Switch  Criteria=u2 ~= 0
  Terminator : Terminator
  Unit Delay{,1..4} (5 alike) : UnitDelay  SampleTime=-1
  signal_filter : Subsystem
  StrAng : Outport  InitialOutput=[0]
  StrAng1 : Outport  Port=2  InitialOutput=[0]
  StrAng2 : Outport  Port=3  InitialOutput=[0]
  StrAng3 : Outport  Port=4  InitialOutput=[0]
  StrAng4 : Outport  Port=5  InitialOutput=[0]
  wires:
  Unit Delay4/1 -> StrAng4/1
  Unit Delay3/1 -> StrAng3/1, Unit Delay4/1
  Unit Delay2/1 -> StrAng2/1, Unit Delay3/1
  Unit Delay1/1 -> StrAng1/1, Unit Delay2/1
  signal_filter/1(raw_input_filt) -> Product/1
  Switch/1 -> Product/2
  Constant1/1 -> Switch/3
  Constant/1 -> Switch/1
  Compare To Zero/1 -> Switch/2
  Product/1 -> Terminator/1  "StrRateRaw_degps"
  Constant3/1 -> Divide/2
  Abs/1 -> Divide/1
  Sum/1 -> Compare To Zero/1, Abs/1
  Unit Delay/1 -> Sum/2
  Constant2/1 -> signal_filter/3(Ts)
  Constant4/1 -> signal_filter/2(Tc)
  Divide/1 -> signal_filter/1(raw_input)  "VINP_SteerSpeedUnFlt_degps"
  In1/1 -> Sum/1, Unit Delay/1, StrAng/1, Unit Delay1/1

== system inp/INP/InputSignals/SignalProcess/Proc_SteerSpeedCal/SteerSpeedCalculation1 ==
  VSTR_StrAngReq_deg : Inport
  Trigger : TriggerPort
  Abs : Abs
  Compare To Zero : simulink/Logic and Bit Operations/Compare To Zero  relop=>=  OutDataTypeStr=boolean
  Constant : Constant  Value=single(1)
  Constant1 : Constant  Value=single(-1)
  Constant2 : Constant  Value=KINP_SteerSpeedCalRate_s
  Constant3 : Constant  Value=KINP_SteerSpeedCalRate_s
  Constant4 : Constant  Value=KINP_SteerSpeedFltConst_s
  Divide : Product  Inputs=*/
  Product : Product
  Sum : Sum  Inputs=+-
  Switch : Switch  Criteria=u2 ~= 0
  Unit Delay : UnitDelay  SampleTime=-1
  signal_filter : Subsystem
  VSTR_StrRateReq_degps : Outport
  wires:
  signal_filter/1(raw_input_filt) -> Product/1
  Switch/1 -> Product/2
  Constant1/1 -> Switch/3
  Constant/1 -> Switch/1
  Compare To Zero/1 -> Switch/2
  Constant3/1 -> Divide/2
  Abs/1 -> Divide/1
  Sum/1 -> Compare To Zero/1, Abs/1
  Unit Delay/1 -> Sum/2
  Constant2/1 -> signal_filter/3(Ts)
  Constant4/1 -> signal_filter/2(Tc)
  Divide/1 -> signal_filter/1(raw_input)
  VSTR_StrAngReq_deg/1 -> Sum/1, Unit Delay/1
  Product/1 -> VSTR_StrRateReq_degps/1

== system inp/INP/InputSignals/SignalProcess/Proc_SteerSpeedCal/Trigger signal generator ==
  Constant3 : Constant  Value=KINP_SteerSpeedCalRate_s
  trigger signal generator : Subsystem  TreatAsAtomicUnit=on
  Trigger_signal : Outport
  wires:
  Constant3/1 -> trigger signal generator/1(Time_dur [s])
  trigger signal generator/1(Trigger_signal) -> Trigger_signal/1

== system inp/INP/InputSignals/SignalProcess/Proc_SteerSpeedCal/SteerSpeedCalculation/signal_filter ==
  raw_input : Inport
  Tc : Inport  Port=2
  Ts : Inport  Port=3
  FirstOrderFilter : hcu_lib/FirstOrderFilter  SystemSampleTime=-1
  IC : UnitDelay  Tag=struct('comment',struct('text','','placement',1),'logdata',struct('maxnumvals',inf,'plotchannels',[],'stepsize',0,'downsampling',1,'loggingmode',3,'channels',1),'output',struct('width',1,'name','$S_$B','unit','','type','Int16','offset',0,'max',NaN,'min',NaN,'freeze',1,'lsb',1,'arb',0,'class','default','address','','description','','autoscalingmode',1,'lowerheadroom',1,'upperheadroom',1,'headroomunit','bit','checkmin',0,'checkmax',0,'show',0,'initial','2'),'state',0,'dt','-1','date',731455.586925799)  SampleTime=-1
  Switch1 : Switch  Tag=struct('comment',struct('text','','placement',1),'logdata',struct('maxnumvals',inf,'plotchannels',[],'stepsize',0,'downsampling',1,'loggingmode',3,'channels',1),'output',struct('width',1,'name','$S_$B','unit','','type','Int32Bin15','offset',0,'max',65535,'min',-65536,'freeze',1,'lsb',2^-15,'arb',0,'class','default','address','','description','','autoscalingmode',1,'lowerheadroom',1,'upperheadroom',1,'headroomunit','bit','checkmin',0,'checkmax',0,'show',0),'threshold',struct('width',1,'name','$S_$B_threshold','unit','','type','Int16','offset',0,'max',NaN,'min',NaN,'freeze',1,'lsb',1,'arb',0,'class','default','address','','description','','value','1','scalar',0),'date',731455.58657044)  Threshold=0.5  InputSameDT=on
  zero : Constant  Tag=struct('comment',struct('text','','placement',1),'output',struct('width',1,'name','$S_$B','unit','','type','Int16','offset',0,'max',NaN,'min',NaN,'freeze',1,'lsb',1,'arb',0,'class','default','address','','description','','value','0','tolerance',1,'show',0),'date',731455.58675544)  Value=TRUE
  zero1 : Constant  Tag=struct('comment',struct('text','','placement',1),'output',struct('width',1,'name','$S_$B','unit','','type','Int16','offset',0,'max',NaN,'min',NaN,'freeze',1,'lsb',1,'arb',0,'class','default','address','','description','','value','0','tolerance',1,'show',0),'date',731455.58675544)  Value=0  OutDataTypeStr=single
  raw_input_filt : Outport
  wires:
  zero/1 -> IC/1
  raw_input/1 -> FirstOrderFilter/1
  Tc/1 -> Switch1/1
  Ts/1 -> FirstOrderFilter/3
  IC/1 -> Switch1/2
  FirstOrderFilter/1 -> raw_input_filt/1
  Switch1/1 -> FirstOrderFilter/2
  zero1/1 -> Switch1/3

== system inp/INP/InputSignals/SignalProcess/Proc_SteerSpeedCal/SteerSpeedCalculation1/signal_filter ==
  raw_input : Inport
  Tc : Inport  Port=2
  Ts : Inport  Port=3
  FirstOrderFilter : hcu_lib/FirstOrderFilter  SystemSampleTime=-1
  IC : UnitDelay  Tag=struct('comment',struct('text','','placement',1),'logdata',struct('maxnumvals',inf,'plotchannels',[],'stepsize',0,'downsampling',1,'loggingmode',3,'channels',1),'output',struct('width',1,'name','$S_$B','unit','','type','Int16','offset',0,'max',NaN,'min',NaN,'freeze',1,'lsb',1,'arb',0,'class','default','address','','description','','autoscalingmode',1,'lowerheadroom',1,'upperheadroom',1,'headroomunit','bit','checkmin',0,'checkmax',0,'show',0,'initial','2'),'state',0,'dt','-1','date',731455.586925799)  SampleTime=-1
  Switch1 : Switch  Tag=struct('comment',struct('text','','placement',1),'logdata',struct('maxnumvals',inf,'plotchannels',[],'stepsize',0,'downsampling',1,'loggingmode',3,'channels',1),'output',struct('width',1,'name','$S_$B','unit','','type','Int32Bin15','offset',0,'max',65535,'min',-65536,'freeze',1,'lsb',2^-15,'arb',0,'class','default','address','','description','','autoscalingmode',1,'lowerheadroom',1,'upperheadroom',1,'headroomunit','bit','checkmin',0,'checkmax',0,'show',0),'threshold',struct('width',1,'name','$S_$B_threshold','unit','','type','Int16','offset',0,'max',NaN,'min',NaN,'freeze',1,'lsb',1,'arb',0,'class','default','address','','description','','value','1','scalar',0),'date',731455.58657044)  Threshold=0.5  InputSameDT=on
  zero : Constant  Tag=struct('comment',struct('text','','placement',1),'output',struct('width',1,'name','$S_$B','unit','','type','Int16','offset',0,'max',NaN,'min',NaN,'freeze',1,'lsb',1,'arb',0,'class','default','address','','description','','value','0','tolerance',1,'show',0),'date',731455.58675544)  Value=TRUE
  zero1 : Constant  Tag=struct('comment',struct('text','','placement',1),'output',struct('width',1,'name','$S_$B','unit','','type','Int16','offset',0,'max',NaN,'min',NaN,'freeze',1,'lsb',1,'arb',0,'class','default','address','','description','','value','0','tolerance',1,'show',0),'date',731455.58675544)  Value=0  OutDataTypeStr=single
  raw_input_filt : Outport
  wires:
  zero/1 -> IC/1
  raw_input/1 -> FirstOrderFilter/1
  Tc/1 -> Switch1/1
  Ts/1 -> FirstOrderFilter/3
  IC/1 -> Switch1/2
  FirstOrderFilter/1 -> raw_input_filt/1
  Switch1/1 -> FirstOrderFilter/2
  zero1/1 -> Switch1/3

== system inp/INP/InputSignals/SignalProcess/Proc_SteerSpeedCal/Trigger signal generator/trigger signal generator ==
  Time_dur [s] : Inport
  Constant3 : Constant  Value=0  OutDataTypeStr=single
  Constant4 : Constant  Value=C_TICK_TIME_S
  Relational Operator1 : RelationalOperator  OutDataTypeStr=boolean
  Sum1 : Sum  Inputs=|++  SaturateOnIntegerOverflow=on  InputSameDT=on
  Sum2 : Sum  Inputs=+-
  Switch3 : Switch  Threshold=0.5
  Unit Delay2 : UnitDelay  SampleTime=-1
  Unit Delay3 : UnitDelay  SampleTime=-1
  Trigger_signal : Outport  OutputWhenDisabled=reset  InitialOutput=[0]
  wires:
  Sum1/1 -> Switch3/3
  Constant4/1 -> Sum1/1, Sum2/2
  Relational Operator1/1 -> Trigger_signal/1, Unit Delay3/1
  Time_dur [s]/1 -> Sum2/1
  Constant3/1 -> Switch3/1
  Switch3/1 -> Unit Delay2/1, Relational Operator1/1
  Unit Delay2/1 -> Sum1/2
  Unit Delay3/1 -> Switch3/2
  Sum2/1 -> Relational Operator1/2

== stateflow chart INP/InputSignals/SignalProcess/Proc_SteerSpeedCal/StrRate_calculation ==
  (default) -> (junction 17)
  (junction 17) -> (junction 18) [order 1] : [CAN_flag]
  (junction 17) -> (junction 21) [order 2]
  (junction 18) -> (junction 19) : {StrRate1=(StrAng-StrAng1)/(1*KINP_SteerSpeedCalRate_s);
      | StrRate2=(StrAng-StrAng2)/(2*KINP_SteerSpeedCalRate_s);
      | StrRate3=(StrAng-StrAng3)/(3*KINP_SteerSpeedCalRate_s);
      | StrRate4=(StrAng-StrAng4)/(4*KINP_SteerSpeedCalRate_s);}
  (junction 19) -> (junction 20) [order 1] : [abs(StrRate1)<=KINP_StrRate_Threshold && abs(StrRate2)<=0.5*KINP_StrRate_Threshold]
  (junction 19) -> (junction 23) [order 2]
  (junction 20) -> (junction 29) : {StrRate=0.25*(StrRate1+StrRate2+StrRate3+StrRate4);}
  (junction 21) -> (junction 22) [order 1] : [abs(StrRate1)<=KINP_StrRate_Threshold && abs(StrRate2)<=0.5*KINP_StrRate_Threshold]
  (junction 21) -> (junction 25) [order 2] : {StrRate=0.5*(StrRateLast+StrRate1);}
  (junction 22) -> (junction 26) : {StrRate=0.333*(StrRate1+StrRate2+StrRate3);}
  (junction 23) -> (junction 24) [order 1] : [abs(StrRateLast-StrRate1)<=abs(StrRateLast-StrRate2)]
  (junction 23) -> (junction 27) [order 2] : {StrRate=StrRate2;}
  (junction 24) -> (junction 28) : {StrRate=StrRate1;}
  (junction 26) -> (junction 25)
  (junction 27) -> (junction 26)
  (junction 28) -> (junction 27)
  (junction 29) -> (junction 28)
  data: CAN_flag input boolean; StrRate1 local single; StrRate2 local single; StrRate3 local single; StrRate output single; KINP_SteerSpeedCalRate_s parameter single; StrAng input single; StrAng1 input single; StrAng2 input single; StrAng3 input single; StrAng4 input single; StrRateLast input single; StrRate4 local single; KINP_StrRate_Threshold parameter double

== stateflow chart INP/InputSignals/SignalProcess/Proc_SteerSpeedCal/CAN_Update_flag ==
  (default) -> (junction 52)
  (junction 52) -> (junction 53) [order 1] : [AngleChg || CAN_flag_prev]
  (junction 52) -> (junction 54) [order 2] : {CAN_flag=0; ...
      | CAN_flag_prev=1;}
  (junction 53) -> (junction 55) : {CAN_flag=1; ...
      | CAN_flag_prev=0;}
  (junction 55) -> (junction 54)
  data: AngleChg input boolean; CAN_flag output boolean; CAN_flag_prev local boolean

== stateflow chart INP/InputSignals/SignalProcess/Proc_OtherHMISwitches/RearFogOnSwitchLatch ==
  state WaitingOn
    en:VINP_RearFogSwOn_flg_InProc=FALSE;
  state RearFogOn
    en:VINP_RearFogSwOn_flg_InProc=TRUE;
  state WaitingOff
    en:VINP_RearFogSwOn_flg_InProc=TRUE;
  state RearFogOff
    en:VINP_RearFogSwOn_flg_InProc=FALSE;
  (default) -> WaitingOn
  WaitingOn -> RearFogOn : [VINP_RearFogSwOn_flg_InHAL]
  RearFogOn -> WaitingOff : [!VINP_RearFogSwOn_flg_InHAL]
  (junction 71) -> WaitingOn
  WaitingOff -> (junction 72)
  RearFogOff -> (junction 71) : [!VINP_RearFogSwOn_flg_InHAL]
  (junction 72) -> RearFogOff : [VINP_RearFogSwOn_flg_InHAL]
  data: VINP_RearFogSwOn_flg_InProc output boolean; VINP_RearFogSwOn_flg_InHAL input double; TRUE parameter double; FALSE parameter double
